simulink model "roachf_2048_bak"  (Simulink 7.5)
settings: Solver=ode45  StartTime=0.0  StopTime=10.0  RelTol=1e-3
undefined symbols (not defined in the file or in its folder): Down, quant, ROACH, Up
libraries: xbsIndex_r4 x1733, xps_library x50, casper_library_misc x29, casper_library_delays x4, casper_library_sources x4, xbsReplacements_r4 x4, simulink x1, xrbsControl_r4 x1
(part 1 of 18)
settings Model: Creator=zaki  LastModifiedBy=zaki
settings DebuggingCC: StrictBusMsg=Warning

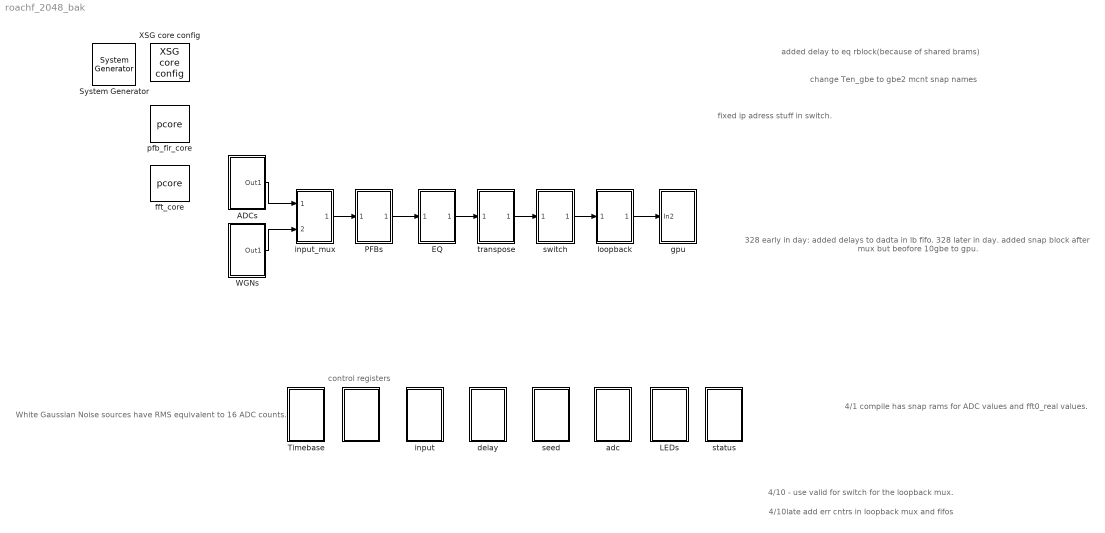
== system roachf_2048_bak ==
  note: White Gaussian Noise
      | sources have RMS
      | equivalent to 16 ADC
      | counts.
  note: fixed ip adress stuff in switch.
  note: 328 early in day: added delays to dadta in lb fifo.
      | 328 later in day. added snap block after mux but beofore
      | 10gbe to gpu.
  note: 4/1 compile has snap rams for ADC values
      | and fft0_real values.
  note: 4/10 - use valid for switch for the loopback mux.
  note: 4/10late add err cntrs in loopback mux and fifos
  note: control registers
  note: added delay to eq rblock(because of shared brams)
  note: change Ten_gbe to gbe2
      | mcnt snap names
  " " : Subsystem
  " System Generator" : xbsIndex_r4/ System Generator  Tag=genX  SystemSampleTime=-1  xilinxfamily=virtex5  part=xc5vsx95t  speed=-1  package=ff1136  synthesis_tool=XST  clock_wrapper=Clock Enables  directory=./roachf_2048_bak/sysgen  proj_type=Project Navigator  Synth_file=XST Defaults  Impl_file=ISE Defaults  testbench=off  simulink_period=1  sysclk_period=5  dcm_input_clock_period=100  incr_netlist=off  trim_vbits=Everywhere in SubSystem  dbl_ovrd=According to Block Masks  core_generation=According to Block Masks  run_coregen=off  deprecated_control=off  eval_field=0  block_type=sysgen
      settings: block_version=10.1.3
  ADCs : Subsystem
  EQ : Subsystem
  LEDs : Subsystem
  PFBs : Subsystem
  Timebase : Subsystem
  WGNs : Subsystem
  XSG core config : xps_library/XSG core config  Tag=xps:xsg  SystemSampleTime=-1  hw_sys=ROACH:sx95t  ibob_linux=off  clk_src=adc0_clk  iBOB_clk_src=adc0_clk  BEE2_ctrl_clk_src=sys_clk  BEE2_usr_clk_src=sys_clk  ROACH_clk_src=adc0_clk  ROACH2_clk_src=adc0_clk  CORR_clk_src=sys_clk  gpio_clk_io_group=iBOB:sma  gpio_clk_bit_index=0  clk_rate=200  sample_period=1  synthesis_tool=XST  mpc_type=powerpc440_ext
  adc : Subsystem
  delay : Subsystem
      settings: AttributesFormatString=|
      | Addr 8195
      | 0x2003
  fft_core : xps_library/pcore  Tag=xps:pcore  SystemSampleTime=-1  pcore_path=fft_core/fft_core.ngc
  gpu : Subsystem
  input : Subsystem
      settings: AttributesFormatString=|
      | Addr 8194
      | 0x2002
  input_mux : Subsystem
  loopback : Subsystem
  pfb_fir_core : xps_library/pcore  Tag=xps:pcore  SystemSampleTime=-1  pcore_path=pfb_fir_core/pfb_fir_core.ngc
  seed : Subsystem
      settings: AttributesFormatString=|
      | Addr 8196
      | 0x2004
  status : Subsystem
  switch : Subsystem
  transpose : Subsystem
  wires:
  ADCs/1(Out1) -> input_mux/1(In1)
  WGNs/1(Out1) -> input_mux/2(In2)
  input_mux/1(Out1) -> PFBs/1(In1)
  PFBs/1(Out1) -> EQ/1(In1)
  EQ/1(Out1) -> transpose/1(In1)
  transpose/1(Out1) -> switch/1(In2)
  switch/1(Out1) -> loopback/1(In1)
  loopback/1(Out1) -> gpu/1(In2)

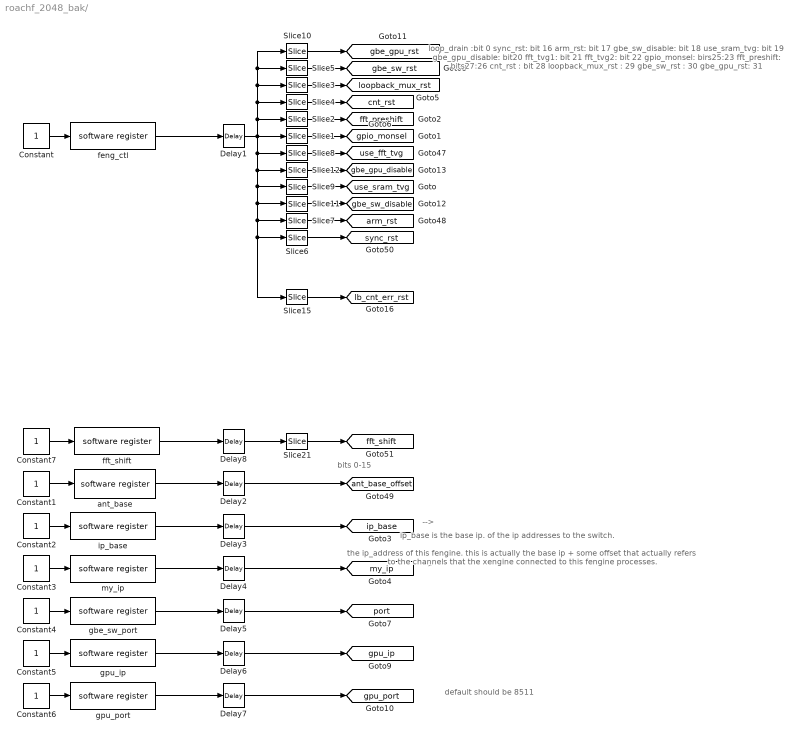
== system roachf_2048_bak/  ==
  note: bits 0-15
  note: default should be 8511
  note: -->
  note: -->
  note: the ip_address of this fengine.
      | this is actually the base ip + some offset
      | that actually refers to the channels that
      | the xengine connected to this fengine
      | processes.
  note: ip_base is the base ip. of
      | the ip addresses to the switch.
  note: loop_drain :bit 0
      |
      | sync_rst: bit 16
      | arm_rst: bit 17
      | gbe_sw_disable: bit 18
      | use_sram_tvg: bit 19
      | gbe_gpu_disable: bit20
      | fft_tvg1: bit 21
      | fft_tvg2: bit 22
      | gpio_monsel: birs25:23
      | fft_preshift: bits27:26
      | cnt_rst : bit 28
      | loopback_mux_rst : 29
      | gbe_sw_rst : 30
      | gbe_gpu_rst: 31
  Constant{,1..7} (8 alike) : Constant
  Delay{1..8} (8 alike) : xbsIndex_r4/Delay  rst=off  infoeditControl=Selection of Reset will increase slice count due to use of real FFs and instead of SRLs  en=off  latency=1  dbl_ovrd=off  reg_retiming=on  block_type=delay
      settings: xl_area=[0, 0, 0, 0, 0, 0, 0]
  Slice1 : xbsIndex_r4/Slice  nbits=3  boolean_output=off  mode=Lower Bit Location + Width  bit1=0  base1=MSB of Input  bit0=23  base0=LSB of Input  dbl_ovrd=off  block_type=slice
      settings: infoedit="Extracts a given range of bits from each input sample and presents it at the output.  The output type is ordinarily unsigned with binary point at zero, but can be Boolean when the slice is one bit wide.<P><P>Hardware notes: In hardware this block costs nothing."  block_version=10.1.3
  Slice10 : xbsIndex_r4/Slice  nbits=16  boolean_output=on  mode=Lower Bit Location + Width  bit1=0  base1=MSB of Input  bit0=31  base0=LSB of Input  dbl_ovrd=off  block_type=slice
      settings: block_version=10.1.3
  Slice11 : xbsIndex_r4/Slice  nbits=1  boolean_output=on  mode=Lower Bit Location + Width  bit1=0  base1=MSB of Input  bit0=18  base0=LSB of Input  dbl_ovrd=off  block_type=slice
      settings: block_version=10.1.3
  Slice12 : xbsIndex_r4/Slice  nbits=1  boolean_output=on  mode=Lower Bit Location + Width  bit1=0  base1=MSB of Input  bit0=20  base0=LSB of Input  dbl_ovrd=off  block_type=slice
      settings: block_version=10.1.3
  Slice15 : xbsIndex_r4/Slice  nbits=1  boolean_output=on  mode=Lower Bit Location + Width  bit1=0  base1=MSB of Input  bit0=2  base0=LSB of Input  dbl_ovrd=off  block_type=slice
      settings: block_version=10.1.3
  Slice2 : xbsIndex_r4/Slice  nbits=2  boolean_output=off  mode=Lower Bit Location + Width  bit1=0  base1=MSB of Input  bit0=26  base0=LSB of Input  dbl_ovrd=off  block_type=slice
      settings: infoedit="Extracts a given range of bits from each input sample and presents it at the output.  The output type is ordinarily unsigned with binary point at zero, but can be Boolean when the slice is one bit wide.<P><P>Hardware notes: In hardware this block costs nothing."  block_version=10.1.3
  Slice21 : xbsIndex_r4/Slice  nbits=16  boolean_output=off  mode=Lower Bit Location + Width  bit1=0  base1=MSB of Input  bit0=0  base0=LSB of Input  dbl_ovrd=off  block_type=slice
      settings: infoedit="Extracts a given range of bits from each input sample and presents it at the output.  The output type is ordinarily unsigned with binary point at zero, but can be Boolean when the slice is one bit wide.<P><P>Hardware notes: In hardware this block costs nothing."  block_version=10.1.3
  Slice3 : xbsIndex_r4/Slice  nbits=16  boolean_output=on  mode=Lower Bit Location + Width  bit1=0  base1=MSB of Input  bit0=29  base0=LSB of Input  dbl_ovrd=off  block_type=slice
      settings: block_version=10.1.3
  Slice4 : xbsIndex_r4/Slice  nbits=16  boolean_output=on  mode=Lower Bit Location + Width  bit1=0  base1=MSB of Input  bit0=28  base0=LSB of Input  dbl_ovrd=off  block_type=slice
      settings: block_version=10.1.3
  Slice5 : xbsIndex_r4/Slice  nbits=16  boolean_output=on  mode=Lower Bit Location + Width  bit1=0  base1=MSB of Input  bit0=30  base0=LSB of Input  dbl_ovrd=off  block_type=slice
      settings: block_version=10.1.3
  Slice6 : xbsIndex_r4/Slice  nbits=1  boolean_output=on  mode=Lower Bit Location + Width  bit1=0  base1=MSB of Input  bit0=16  base0=LSB of Input  dbl_ovrd=off  block_type=slice
      settings: infoedit="Extracts a given range of bits from each input sample and presents it at the output.  The output type is ordinarily unsigned with binary point at zero, but can be Boolean when the slice is one bit wide.<P><P>Hardware notes: In hardware this block costs nothing."  block_version=10.1.3
  Slice7 : xbsIndex_r4/Slice  nbits=1  boolean_output=on  mode=Lower Bit Location + Width  bit1=0  base1=MSB of Input  bit0=17  base0=LSB of Input  dbl_ovrd=off  block_type=slice
      settings: infoedit="Extracts a given range of bits from each input sample and presents it at the output.  The output type is ordinarily unsigned with binary point at zero, but can be Boolean when the slice is one bit wide.<P><P>Hardware notes: In hardware this block costs nothing."  block_version=10.1.3
  Slice8 : xbsIndex_r4/Slice  nbits=2  boolean_output=off  mode=Lower Bit Location + Width  bit1=0  base1=MSB of Input  bit0=21  base0=LSB of Input  dbl_ovrd=off  block_type=slice
      settings: infoedit="Extracts a given range of bits from each input sample and presents it at the output.  The output type is ordinarily unsigned with binary point at zero, but can be Boolean when the slice is one bit wide.<P><P>Hardware notes: In hardware this block costs nothing."  block_version=10.1.3
  Slice9 : xbsIndex_r4/Slice  nbits=1  boolean_output=off  mode=Lower Bit Location + Width  bit1=0  base1=MSB of Input  bit0=19  base0=LSB of Input  dbl_ovrd=off  block_type=slice
      settings: infoedit="Extracts a given range of bits from each input sample and presents it at the output.  The output type is ordinarily unsigned with binary point at zero, but can be Boolean when the slice is one bit wide.<P><P>Hardware notes: In hardware this block costs nothing."  block_version=10.1.3
  ant_base : xps_library/software register  Tag=xps:sw_reg  SystemSampleTime=-1  io_dir=From Processor  arith_type=Unsigned  bitwidth=32  bin_pt=0  sample_period=1  gw_name=roachf_2048_bak_ant_base_user_data_out
  feng_ctl : xps_library/software register  Tag=xps:sw_reg  SystemSampleTime=-1  io_dir=From Processor  arith_type=Unsigned  bitwidth=32  bin_pt=0  sample_period=1  gw_name=roachf_2048_bak_feng_ctl_user_data_out
  fft_shift : xps_library/software register  Tag=xps:sw_reg  SystemSampleTime=-1  io_dir=From Processor  arith_type=Unsigned  bitwidth=32  bin_pt=0  sample_period=1  gw_name=roachf_2048_bak_fft_shift_user_data_out
  gbe_sw_port : xps_library/software register  Tag=xps:sw_reg  SystemSampleTime=-1  io_dir=From Processor  arith_type=Unsigned  bitwidth=32  bin_pt=0  sample_period=1  gw_name=roachf_2048_bak_gbe_sw_port_user_data_out
  gpu_ip : xps_library/software register  Tag=xps:sw_reg  SystemSampleTime=-1  io_dir=From Processor  arith_type=Unsigned  bitwidth=32  bin_pt=0  sample_period=1  gw_name=roachf_2048_bak_gpu_ip_user_data_out
  gpu_port : xps_library/software register  Tag=xps:sw_reg  SystemSampleTime=-1  io_dir=From Processor  arith_type=Unsigned  bitwidth=32  bin_pt=0  sample_period=1  gw_name=roachf_2048_bak_gpu_port_user_data_out
  ip_base : xps_library/software register  Tag=xps:sw_reg  SystemSampleTime=-1  io_dir=From Processor  arith_type=Unsigned  bitwidth=32  bin_pt=0  sample_period=1  gw_name=roachf_2048_bak_ip_base_user_data_out
  my_ip : xps_library/software register  Tag=xps:sw_reg  SystemSampleTime=-1  io_dir=From Processor  arith_type=Unsigned  bitwidth=32  bin_pt=0  sample_period=1  gw_name=roachf_2048_bak_my_ip_user_data_out
  tag scope: ant_base_offset=global, arm_rst=global, cnt_rst=global, fft_preshift=global, fft_shift=global, gbe_gpu_disable=global, gbe_gpu_rst=global, gbe_sw_disable=global, gbe_sw_rst=global, gpio_monsel=global, gpu_ip=global, gpu_port=global, ip_base=global, lb_cnt_err_rst=global, loopback_mux_rst=global, my_ip=global, port=global, sync_rst=global, use_fft_tvg=global, use_sram_tvg=global
  wires:
  Slice15/1 -> [lb_cnt_err_rst]
  Delay8/1 -> Slice21/1
  Constant7/1 -> fft_shift/1
  fft_shift/1 -> Delay8/1
  Slice12/1 -> [gbe_gpu_disable]
  Slice11/1 -> [gbe_sw_disable]
  Slice10/1 -> [gbe_gpu_rst]
  gpu_port/1 -> Delay7/1
  Delay7/1 -> [gpu_port]
  Constant6/1 -> gpu_port/1
  gpu_ip/1 -> Delay6/1
  Delay6/1 -> [gpu_ip]
  Constant5/1 -> gpu_ip/1
  Slice5/1 -> [gbe_sw_rst]
  gbe_sw_port/1 -> Delay5/1
  Delay5/1 -> [port]
  Constant4/1 -> gbe_sw_port/1
  Slice3/1 -> [loopback_mux_rst]
  Slice4/1 -> [cnt_rst]
  my_ip/1 -> Delay4/1
  Delay4/1 -> [my_ip]
  Constant3/1 -> my_ip/1
  ip_base/1 -> Delay3/1
  Delay3/1 -> [ip_base]
  Constant2/1 -> ip_base/1
  Constant1/1 -> ant_base/1
  Constant/1 -> feng_ctl/1
  feng_ctl/1 -> Delay1/1
  Slice2/1 -> [fft_preshift]
  Slice1/1 -> [gpio_monsel]
  Slice9/1 -> [use_sram_tvg]
  Slice7/1 -> [arm_rst]
  Slice6/1 -> [sync_rst]
  Slice21/1 -> [fft_shift]
  Delay1/1 -> Slice10/1, Slice5/1, Slice3/1, Slice4/1, Slice2/1, Slice1/1, Slice12/1, Slice9/1, Slice11/1, Slice15/1, Slice6/1, Slice7/1, Slice8/1
  Slice8/1 -> [use_fft_tvg]
  ant_base/1 -> Delay2/1
  Delay2/1 -> [ant_base_offset]

== system roachf_2048_bak/ADCs ==
  Band-Limited White Noise : simulink/Sources/Band-Limited White Noise  SystemSampleTime=-1  Cov=[0.1]  Ts=0.1  seed=[23341]
  Constant : Constant  Value=0
  Constant1 : Constant
  Constant2 : Constant  Value=0
  Mux : Mux  Inputs=2
  Pulse Generator : DiscretePulseGenerator  Amplitude=1  Period=2^27  PulseWidth=1  PhaseDelay=0  SampleTime=1
  Terminator11 : Terminator
  Terminator12 : Terminator
  quadc0 : xps_library/quadc  Tag=xps:quadc  SystemSampleTime=-1  adc_brd=0  adc_clk_rate=200
  quadc1 : xps_library/quadc  Tag=xps:quadc  SystemSampleTime=-1  adc_brd=1  adc_clk_rate=200
  Out1 : Outport
  tag scope: adc0=global, adc1=global, adc2=global, adc3=global, adc4=global, adc5=global, adc6=global, adc7=global, sync_adc0=global, sync_adc1=global
  wires:
  quadc0/1 -> [adc0]
  quadc0/2 -> [adc1]
  quadc0/3 -> [adc2]
  quadc0/4 -> [adc3]
  quadc0/6 -> [sync_adc0]
  quadc1/1 -> [adc4]
  quadc1/2 -> [adc5]
  quadc1/3 -> [adc6]
  quadc1/4 -> [adc7]
  quadc1/6 -> [sync_adc1]
  Band-Limited White Noise/1 -> quadc1/4, quadc1/3, quadc1/2, quadc1/1, quadc0/4, quadc0/3, quadc0/2, quadc0/1
  Pulse Generator/1 -> quadc0/6, quadc1/6
  Constant1/1 -> quadc0/5, quadc1/5
  quadc0/5 -> Terminator11/1
  quadc1/5 -> Terminator12/1
  Mux/1 -> Out1/1
  Constant/1 -> Mux/1
  Constant2/1 -> Mux/2

== system roachf_2048_bak/EQ ==
  note: Next, we reduce the bitwidth out of the FFT to 4 bits to save resources.
      | This must be done very carefully to avoid losing too much information.
      | Firstly, this conversion is done using "Round to Even" and saturation
      | logic to avoid introducing DC offsets, wrapping, and other ugly quantizaton
      | effects.  Secondly, the "equalizer" allows each frequency channel of the
      | FFT to be scaled by a different number.  This allows passbands which are
      | not flat to be flattened digitally to allow for optimal quantization to 4 bits.
      | These coefficients can also be updated dynamically for gain control.
      | Coefficients can be dynamically written into the "equalizer" by
      | specifying the channel ("coeff_addr") and the value of the
      | coefficient ("coeff"), and then using "coeff_en" to write the
      | new coefficient into the equalization table.
  note: eq_mon will monitor eq0, which consists of
      | in0 spectrum followed by in1 spectrum
  In1 : Inport
  Concat3 : xbsIndex_r4/Concat  num_inputs=4  dbl_ovrd=off  block_type=concat
      settings: block_version=10.1.3
  Logical5 : xbsIndex_r4/Logical  logical_function=OR  inputs=4  en=off  latency=0  precision=Full  arith_type=Unsigned  n_bits=8  bin_pt=2  align_bp=on  dbl_ovrd=off  block_type=logical
      settings: xl_area=[0, 0, 0, 0, 0, 0, 0]
  Terminator13 : Terminator
  Terminator14 : Terminator
  Terminator15 : Terminator
  eq0 : Subsystem
  eq1 : Subsystem
  eq2 : Subsystem
  eq3 : Subsystem
  Out1 : Outport
  tag scope: eq0=global, eq1=global, eq2=global, eq3=global, eq_clip0=global, eq_clip1=global, eq_clip2=global, eq_clip3=global, eq_clip=global, eq_mon=global, eq_out=global, sync_rnd=global
  wires:
  eq0/1(dout) -> [eq0], [eq_mon]
  eq0/2(sync_out) -> [sync_rnd]
  [fft0] -> eq0/1(in)
  eq0/3(clip) -> [eq_clip0]
  Logical5/1 -> [eq_clip]
  Concat3/1 -> [eq_out]
  [eq_clip0] -> Logical5/1
  [eq_clip1] -> Logical5/2
  [fft1] -> eq1/1(in)
  [sync_fft0] -> eq1/2(sync)
  [fft2] -> eq2/1(in)
  [fft3] -> eq3/1(in)
  [sync_fft0] -> eq3/2(sync)
  eq1/1(dout) -> [eq1]
  eq1/2(sync_out) -> Terminator13/1
  eq1/3(clip) -> [eq_clip1]
  eq2/1(dout) -> [eq2]
  eq2/2(sync_out) -> Terminator14/1
  eq2/3(clip) -> [eq_clip2]
  eq3/1(dout) -> [eq3]
  eq3/2(sync_out) -> Terminator15/1
  eq3/3(clip) -> [eq_clip3]
  [eq_clip2] -> Logical5/3
  [eq_clip3] -> Logical5/4
  [eq0] -> Concat3/1
  [eq1] -> Concat3/2
  [eq2] -> Concat3/3
  [eq3] -> Concat3/4
  In1/1 -> Out1/1
  [sync_fft0] -> eq0/2(sync)
  [sync_fft0] -> eq2/2(sync)

== system roachf_2048_bak/LEDs ==
  Delay{,1..7} (8 alike) : xbsIndex_r4/Delay  rst=off  infoeditControl=Selection of Reset will increase slice count due to use of real FFs and instead of SRLs  en=off  latency=1  dbl_ovrd=off  reg_retiming=off  block_type=delay
  Terminator{1..3,27} (4 alike) : Terminator
  led1_arm : xps_library/gpio  Tag=xps:gpio  SystemSampleTime=-1  io_group=ROACH:led  io_dir=out  arith_type=Boolean  bitwidth=1  bin_pt=0  bit_index=0  sample_period=1  use_single_ended=off  use_ddr=off  reg_iob=on  reg_clk_phase=0  termination=None
      link override convert: arith_type=Boolean  n_bits=1  bin_pt=0
  led2_sync : xps_library/gpio  Tag=xps:gpio  SystemSampleTime=-1  io_group=ROACH:led  io_dir=out  arith_type=Boolean  bitwidth=1  bin_pt=0  bit_index=1  sample_period=1  use_single_ended=off  use_ddr=off  reg_iob=on  reg_clk_phase=0  termination=None
      link override convert: arith_type=Boolean  n_bits=1  bin_pt=0
  led4_eq_clip : xps_library/gpio  Tag=xps:gpio  SystemSampleTime=-1  io_group=ROACH:led  io_dir=out  arith_type=Boolean  bitwidth=1  bin_pt=0  bit_index=2  sample_period=1  use_single_ended=off  use_ddr=off  reg_iob=on  reg_clk_phase=0  termination=None
      link override convert: arith_type=Boolean  n_bits=1  bin_pt=0
  led5_adc_clip : xps_library/gpio  Tag=xps:gpio  SystemSampleTime=-1  io_group=ROACH:led  io_dir=out  arith_type=Boolean  bitwidth=1  bin_pt=0  bit_index=3  sample_period=1  use_single_ended=off  use_ddr=off  reg_iob=on  reg_clk_phase=0  termination=None
      link override convert: arith_type=Boolean  n_bits=1  bin_pt=0
  pulse_ext2 : Subsystem  mask{pulse_len=2^23}
      mask prompts: pulse_len "Length of Pulse"
      mask init:
      | bits = ceil(log2(pulse_len+1));
  pulse_ext3 : Subsystem  mask{pulse_len=2^18}
      mask prompts: pulse_len "Length of Pulse"
      mask init:
      | bits = ceil(log2(pulse_len+1));
  pulse_ext4 : Subsystem  mask{pulse_len=2^18}
      mask prompts: pulse_len "Length of Pulse"
      mask init:
      | bits = ceil(log2(pulse_len+1));
  wires:
  pulse_ext2/1(out) -> Delay5/1
  [sync_gen] -> Delay1/1
  [armed] -> Delay/1
  pulse_ext4/1(out) -> Delay6/1
  [eq_clip] -> Delay2/1
  led1_arm/1 -> Terminator27/1
  led4_eq_clip/1 -> Terminator2/1
  led2_sync/1 -> Terminator3/1
  [adc_clip] -> Delay3/1
  pulse_ext3/1(out) -> Delay7/1
  led5_adc_clip/1 -> Terminator1/1
  Delay/1 -> Delay4/1
  Delay3/1 -> pulse_ext3/1(in)
  Delay2/1 -> pulse_ext4/1(in)
  Delay1/1 -> pulse_ext2/1(in)
  Delay7/1 -> led5_adc_clip/1
  Delay6/1 -> led4_eq_clip/1
  Delay5/1 -> led2_sync/1
  Delay4/1 -> led1_arm/1

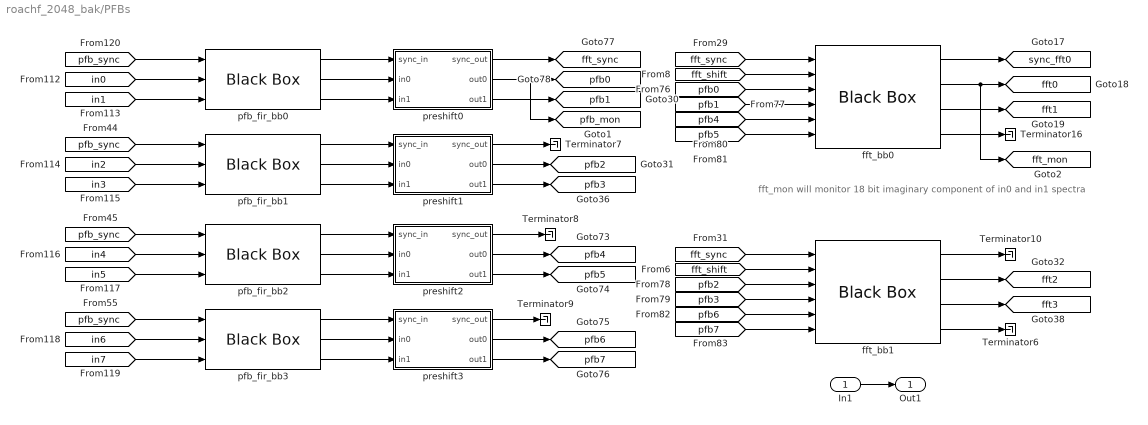
== system roachf_2048_bak/PFBs ==
  note: fft_mon will monitor 18 bit imaginary component of in0 and in1 spectra
  In1 : Inport
  Terminator{6..10,16} (6 alike) : Terminator
  fft_bb0 : xbsIndex_r4/Black Box  init_code=fft_core_config  sim_method=Inactive  verbose=off  block_type=blackbox2
      settings: infoedit="Incorporates black box HDL and simulation model into a System Generator design.<P><P>You must supply a Black Box with certain information about the HDL component you would like to bring into System Generator. This information is provided through a Matlab function.<P><P>When \"Simulation mode\" is set to \"Inactive\", you will typically want to provide a separate simulation model by using a Simulation Multiplexer.<P>When \"Simulation mode\" is set to \"External co-simulator\", you must include a ModelSim block in the design."  block_version=10.1.3
  fft_bb1 : xbsIndex_r4/Black Box  init_code=fft_core_config  sim_method=Inactive  verbose=off  block_type=blackbox2
      settings: infoedit="Incorporates black box HDL and simulation model into a System Generator design.<P><P>You must supply a Black Box with certain information about the HDL component you would like to bring into System Generator. This information is provided through a Matlab function.<P><P>When \"Simulation mode\" is set to \"Inactive\", you will typically want to provide a separate simulation model by using a Simulation Multiplexer.<P>When \"Simulation mode\" is set to \"External co-simulator\", you must include a ModelSim block in the design."  block_version=10.1.3
  pfb_fir_bb{0..3} (4 alike) : xbsIndex_r4/Black Box  init_code=pfb_fir_core_config  sim_method=Inactive  verbose=off  block_type=blackbox2
      settings: infoedit="Incorporates black box HDL and simulation model into a System Generator design.<P><P>You must supply a Black Box with certain information about the HDL component you would like to bring into System Generator. This information is provided through a Matlab function.<P><P>When \"Simulation mode\" is set to \"Inactive\", you will typically want to provide a separate simulation model by using a Simulation Multiplexer.<P>When \"Simulation mode\" is set to \"External co-simulator\", you must include a ModelSim block in the design."  block_version=10.1.3
  preshift0 : Subsystem
  preshift1 : Subsystem
  preshift2 : Subsystem
  preshift3 : Subsystem
  Out1 : Outport
  tag scope: fft0=global, fft1=global, fft2=global, fft3=global, fft_mon=global, fft_sync=global, pfb0=global, pfb1=global, pfb2=global, pfb3=global, pfb4=global, pfb5=global, pfb6=global, pfb7=global, pfb_mon=global, sync_fft0=global
  wires:
  [in0] -> pfb_fir_bb0/2
  [in1] -> pfb_fir_bb0/3
  [in2] -> pfb_fir_bb1/2
  [in3] -> pfb_fir_bb1/3
  [in4] -> pfb_fir_bb2/2
  [in5] -> pfb_fir_bb2/3
  [in6] -> pfb_fir_bb3/2
  [in7] -> pfb_fir_bb3/3
  pfb_fir_bb1/2 -> preshift1/2(in0)
  pfb_fir_bb1/3 -> preshift1/3(in1)
  pfb_fir_bb2/2 -> preshift2/2(in0)
  pfb_fir_bb2/3 -> preshift2/3(in1)
  pfb_fir_bb3/2 -> preshift3/2(in0)
  pfb_fir_bb3/3 -> preshift3/3(in1)
  [fft_sync] -> fft_bb1/1
  [fft_shift] -> fft_bb1/2
  fft_bb1/3 -> [fft3]
  fft_bb1/4 -> Terminator6/1
  fft_bb1/2 -> [fft2]
  fft_bb0/2 -> [fft0], [fft_mon]
  fft_bb0/3 -> [fft1]
  fft_bb0/4 -> Terminator16/1
  fft_bb0/1 -> [sync_fft0]
  fft_bb1/1 -> Terminator10/1
  [fft_sync] -> fft_bb0/1
  [fft_shift] -> fft_bb0/2
  [pfb_sync] -> pfb_fir_bb0/1
  [pfb_sync] -> pfb_fir_bb1/1
  [pfb_sync] -> pfb_fir_bb2/1
  [pfb_sync] -> pfb_fir_bb3/1
  pfb_fir_bb1/1 -> preshift1/1(sync_in)
  pfb_fir_bb2/1 -> preshift2/1(sync_in)
  pfb_fir_bb3/1 -> preshift3/1(sync_in)
  In1/1 -> Out1/1
  pfb_fir_bb0/1 -> preshift0/1(sync_in)
  pfb_fir_bb0/2 -> preshift0/2(in0)
  pfb_fir_bb0/3 -> preshift0/3(in1)
  preshift0/1(sync_out) -> [fft_sync]
  preshift0/2(out0) -> [pfb0], [pfb_mon]
  preshift0/3(out1) -> [pfb1]
  preshift1/1(sync_out) -> Terminator7/1
  preshift2/1(sync_out) -> Terminator8/1
  preshift3/1(sync_out) -> Terminator9/1
  preshift1/2(out0) -> [pfb2]
  preshift1/3(out1) -> [pfb3]
  preshift2/2(out0) -> [pfb4]
  preshift2/3(out1) -> [pfb5]
  preshift3/2(out0) -> [pfb6]
  preshift3/3(out1) -> [pfb7]
  [pfb0] -> fft_bb0/3
  [pfb1] -> fft_bb0/4
  [pfb4] -> fft_bb0/5
  [pfb5] -> fft_bb0/6
  [pfb2] -> fft_bb1/3
  [pfb3] -> fft_bb1/4
  [pfb6] -> fft_bb1/5
  [pfb7] -> fft_bb1/6

== system roachf_2048_bak/Timebase ==
  note: This circuit implements a scheme for synchronizing
      | multiple IBOBs to within a 200 MHz clock.  "arm_rst"
      | comes from the "ctrl" register, and indicates that the
      | next "sync_rst" should reset the sync pulse generated
      | in "sync_gen".  "sync_rst" may be generated from
      | the "ctrl" register, but more often, a 1PPS signal is
      | supplied to the "sync" input of an ADC for this
      | purpose.  Once a reset occurs, "sync_gen" generates
      | a single pulse every 2^27 clocks.  LEDs provide visual
      | confirmation that an arm and reset occur, and "mrst"
      | is passed over XAUI for the purpose of informing
      | downstream logic in a packetized correlator that
      | a "master reset" has occurred.
  Logical7 : xbsIndex_r4/Logical  logical_function=OR  inputs=3  en=off  latency=1  precision=Full  arith_type=Unsigned  n_bits=8  bin_pt=2  align_bp=on  dbl_ovrd=off  block_type=logical
      settings: xl_area=[0, 0, 0, 0, 0, 0, 0]
  sync_gen : Subsystem
  tag scope: armed=global, mrst=global, sync_gen=global
  wires:
  sync_gen/1(sync) -> [sync_gen]
  sync_gen/2(mrst) -> [mrst]
  [arm_rst] -> sync_gen/2(arm)
  [sync_rst] -> Logical7/2
  sync_gen/3(armed) -> [armed]
  Logical7/1 -> sync_gen/1(rst)
  [sync_adc0] -> Logical7/1
  [sync_adc1] -> Logical7/3

== system roachf_2048_bak/WGNs ==
  Constant : Constant  Value=0
  Constant2 : Constant  Value=0
  Mux : Mux  Inputs=2
  noise0 : Subsystem
  noise1 : Subsystem
  noise2 : Subsystem
  noise3 : Subsystem
  Out1 : Outport
  tag scope: n00=global, n01=global, n10=global, n11=global, n20=global, n21=global, n30=global, n31=global
  wires:
  noise0/1(n1) -> [n00]
  noise0/2(n2) -> [n01]
  noise1/1(n1) -> [n10]
  noise1/2(n2) -> [n11]
  noise2/1(n1) -> [n20]
  noise2/2(n2) -> [n21]
  noise3/1(n1) -> [n30]
  noise3/2(n2) -> [n31]
  [seed0] -> noise0/1(seed)
  [seed3] -> noise3/1(seed)
  [seed2] -> noise2/1(seed)
  [seed1] -> noise1/1(seed)
  [seed_ld] -> noise0/2(ld)
  [seed_ld] -> noise3/2(ld)
  [seed_ld] -> noise2/2(ld)
  [seed_ld] -> noise1/2(ld)
  Mux/1 -> Out1/1
  Constant/1 -> Mux/1
  Constant2/1 -> Mux/2

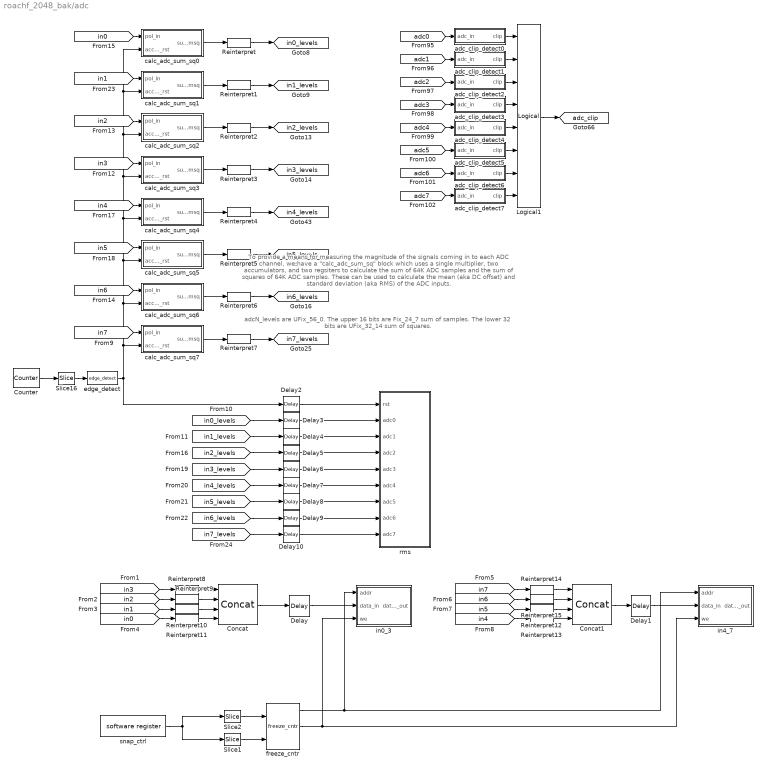
== system roachf_2048_bak/adc ==
  note: To provide a means for measuring the magnitude of the signals
      | coming in to each ADC channel, we have a "calc_adc_sum_sq"
      | block which uses a single multiplier, two accumulators, and two
      | regsiters to calculate the sum of 64K ADC samples and the sum
      | of squares of 64K ADC samples.  These can be used to calculate
      | the mean (aka DC offset) and standard deviation (aka RMS) of
      | the ADC inputs.
  note: adcN_levels are UFix_56_0.
      | The upper 16 bits are Fix_24_7 sum of samples.
      | The lower 32 bits are UFix_32_14 sum of squares.
  Concat : xbsIndex_r4/Concat  num_inputs=4  dbl_ovrd=off  block_type=concat
  Concat1 : xbsIndex_r4/Concat  num_inputs=4  dbl_ovrd=off  block_type=concat
  Counter : xbsIndex_r4/Counter  cnt_type=Free Running  cnt_to=Inf  operation=Up  start_count=0  cnt_by_val=1  arith_type=Unsigned  n_bits=16  bin_pt=0  load_pin=off  rst=off  en=off  explicit_period=off  period=1  dbl_ovrd=off  use_behavioral_HDL=off  implementation=Fabric  use_rpm=on  block_type=counter
      settings: xl_area=[0, 0, 0, 0, 0, 0, 0]
  Delay : xbsIndex_r4/Delay  rst=off  infoeditControl=Selection of Reset will increase slice count due to use of real FFs and instead of SRLs  en=off  latency=3  dbl_ovrd=off  reg_retiming=off  block_type=delay
  Delay1 : xbsIndex_r4/Delay  rst=off  infoeditControl=Selection of Reset will increase slice count due to use of real FFs and instead of SRLs  en=off  latency=3  dbl_ovrd=off  reg_retiming=off  block_type=delay
  Delay{2..10} (9 alike) : xbsIndex_r4/Delay  rst=off  infoeditControl=Selection of Reset will increase slice count due to use of real FFs and instead of SRLs  en=off  latency=2  dbl_ovrd=off  reg_retiming=off  block_type=delay
  Logical1 : xbsIndex_r4/Logical  logical_function=OR  inputs=8  en=off  latency=1  precision=Full  arith_type=Unsigned  n_bits=8  bin_pt=2  align_bp=on  dbl_ovrd=off  block_type=logical
      settings: xl_area=[0, 0, 0, 0, 0, 0, 0]
  Reinterpret{,1..15} (16 alike) : xbsIndex_r4/Reinterpret  force_arith_type=on  arith_type=Unsigned  force_bin_pt=on  bin_pt=0  block_type=reinterpret
      Reinterpret settings: infoedit="Changes signal type without altering the binary representation.   You can changed the signal between signed and unsigned, and relocate the binary point.<P><P>Hardware notes: In hardware this block costs nothing.<P><P>Example:  Suppose the input is 6 bits wide, signed, with 2 fractional bits, and the output is forced to unsigned with 0 fractional bits.  Then an input of -2.0 (1110.00 in binary 2's complement) becomes an output of 56 (111000 in binary)."  block_version=10.1.3
      Reinterpret1 settings: infoedit="Changes signal type without altering the binary representation.   You can changed the signal between signed and unsigned, and relocate the binary point.<P><P>Hardware notes: In hardware this block costs nothing.<P><P>Example:  Suppose the input is 6 bits wide, signed, with 2 fractional bits, and the output is forced to unsigned with 0 fractional bits.  Then an input of -2.0 (1110.00 in binary 2's complement) becomes an output of 56 (111000 in binary)."  block_version=10.1.3
      Reinterpret10 settings: block_version=10.1.3
      Reinterpret11 settings: block_version=10.1.3
      Reinterpret12 settings: block_version=10.1.3
      Reinterpret13 settings: block_version=10.1.3
      Reinterpret14 settings: block_version=10.1.3
      Reinterpret15 settings: block_version=10.1.3
      Reinterpret2 settings: infoedit="Changes signal type without altering the binary representation.   You can changed the signal between signed and unsigned, and relocate the binary point.<P><P>Hardware notes: In hardware this block costs nothing.<P><P>Example:  Suppose the input is 6 bits wide, signed, with 2 fractional bits, and the output is forced to unsigned with 0 fractional bits.  Then an input of -2.0 (1110.00 in binary 2's complement) becomes an output of 56 (111000 in binary)."  block_version=10.1.3
      Reinterpret3 settings: infoedit="Changes signal type without altering the binary representation.   You can changed the signal between signed and unsigned, and relocate the binary point.<P><P>Hardware notes: In hardware this block costs nothing.<P><P>Example:  Suppose the input is 6 bits wide, signed, with 2 fractional bits, and the output is forced to unsigned with 0 fractional bits.  Then an input of -2.0 (1110.00 in binary 2's complement) becomes an output of 56 (111000 in binary)."  block_version=10.1.3
      Reinterpret4 settings: infoedit="Changes signal type without altering the binary representation.   You can changed the signal between signed and unsigned, and relocate the binary point.<P><P>Hardware notes: In hardware this block costs nothing.<P><P>Example:  Suppose the input is 6 bits wide, signed, with 2 fractional bits, and the output is forced to unsigned with 0 fractional bits.  Then an input of -2.0 (1110.00 in binary 2's complement) becomes an output of 56 (111000 in binary)."  block_version=10.1.3
      Reinterpret5 settings: infoedit="Changes signal type without altering the binary representation.   You can changed the signal between signed and unsigned, and relocate the binary point.<P><P>Hardware notes: In hardware this block costs nothing.<P><P>Example:  Suppose the input is 6 bits wide, signed, with 2 fractional bits, and the output is forced to unsigned with 0 fractional bits.  Then an input of -2.0 (1110.00 in binary 2's complement) becomes an output of 56 (111000 in binary)."  block_version=10.1.3
      Reinterpret6 settings: infoedit="Changes signal type without altering the binary representation.   You can changed the signal between signed and unsigned, and relocate the binary point.<P><P>Hardware notes: In hardware this block costs nothing.<P><P>Example:  Suppose the input is 6 bits wide, signed, with 2 fractional bits, and the output is forced to unsigned with 0 fractional bits.  Then an input of -2.0 (1110.00 in binary 2's complement) becomes an output of 56 (111000 in binary)."  block_version=10.1.3
      Reinterpret7 settings: infoedit="Changes signal type without altering the binary representation.   You can changed the signal between signed and unsigned, and relocate the binary point.<P><P>Hardware notes: In hardware this block costs nothing.<P><P>Example:  Suppose the input is 6 bits wide, signed, with 2 fractional bits, and the output is forced to unsigned with 0 fractional bits.  Then an input of -2.0 (1110.00 in binary 2's complement) becomes an output of 56 (111000 in binary)."  block_version=10.1.3
      Reinterpret8 settings: block_version=10.1.3
      Reinterpret9 settings: block_version=10.1.3
  Slice1 : xbsIndex_r4/Slice  nbits=1  boolean_output=on  mode=Lower Bit Location + Width  bit1=0  base1=MSB of Input  bit0=0  base0=LSB of Input  dbl_ovrd=off  block_type=slice
      settings: block_version=10.1.3
  Slice16 : xbsIndex_r4/Slice  nbits=1  boolean_output=on  mode=Upper Bit Location + Width  bit1=0  base1=MSB of Input  bit0=19  base0=LSB of Input  dbl_ovrd=off  block_type=slice
      settings: infoedit="Extracts a given range of bits from each input sample and presents it at the output.  The output type is ordinarily unsigned with binary point at zero, but can be Boolean when the slice is one bit wide.<P><P>Hardware notes: In hardware this block costs nothing."  block_version=10.1.3
  Slice2 : xbsIndex_r4/Slice  nbits=1  boolean_output=on  mode=Lower Bit Location + Width  bit1=0  base1=MSB of Input  bit0=1  base0=LSB of Input  dbl_ovrd=off  block_type=slice
      settings: block_version=10.1.3
  adc_clip_detect0 : Subsystem  mask{n_bits=8}
      mask prompts: n_bits "Number of ADC bits"
  adc_clip_detect1 : Subsystem  mask{n_bits=8}
      mask prompts: n_bits "Number of ADC bits"
  adc_clip_detect2 : Subsystem  mask{n_bits=8}
      mask prompts: n_bits "Number of ADC bits"
  adc_clip_detect3 : Subsystem  mask{n_bits=8}
      mask prompts: n_bits "Number of ADC bits"
  adc_clip_detect4 : Subsystem  mask{n_bits=8}
      mask prompts: n_bits "Number of ADC bits"
  adc_clip_detect5 : Subsystem  mask{n_bits=8}
      mask prompts: n_bits "Number of ADC bits"
  adc_clip_detect6 : Subsystem  mask{n_bits=8}
      mask prompts: n_bits "Number of ADC bits"
  adc_clip_detect7 : Subsystem  mask{n_bits=8}
      mask prompts: n_bits "Number of ADC bits"
  calc_adc_sum_sq0 : Subsystem
  calc_adc_sum_sq1 : Subsystem
  calc_adc_sum_sq2 : Subsystem
  calc_adc_sum_sq3 : Subsystem
  calc_adc_sum_sq4 : Subsystem
  calc_adc_sum_sq5 : Subsystem
  calc_adc_sum_sq6 : Subsystem
  calc_adc_sum_sq7 : Subsystem
  edge_detect : casper_library_misc/edge_detect  SystemSampleTime=-1  edge=Rising  polarity=Active High  x_in=[0 0.08 0.08 0.16 0.16 0.24 0.24 0.32 0.32 0.4]  y_in="[-1 -1  1  1  1  1  1  1  1  1]"  x_out=[0.6 0.68 0.68 0.76 0.76 0.84 0.84 0.92 0.92 1]  y_out="[-1 -1  1  1 -1 -1 -1 -1 -1 -1]"
  freeze_cntr : casper_library_misc/freeze_cntr  SystemSampleTime=-1  CounterBits=10
  in0_3 : Subsystem  Tag=xps:bram  AncestorBlock=xps_library/Shared BRAM  mask{arith_type=Unsigned, addr_width=10, data_width=32, data_bin_pt=0, init_vals=[0:2^10-1], sample_rate=1}
      mask prompts: arith_type "Output Data Type"; addr_width "Address width"; data_width "Data Width"; data_bin_pt "Data Binary Point"; init_vals "Initial 32-bit values (simulation only)"; sample_rate "Sample rate"
      mask init:
      | shared_bram_mask;
      mask StyleString: popup(Unsigned|Signed  (2's comp)),edit,popup(8|16|32|64|128),edit,edit,edit
  in4_7 : Subsystem  Tag=xps:bram  AncestorBlock=xps_library/Shared BRAM  mask{arith_type=Unsigned, addr_width=10, data_width=32, data_bin_pt=0, init_vals=[0:2^10-1], sample_rate=1}
      mask prompts: arith_type "Output Data Type"; addr_width "Address width"; data_width "Data Width"; data_bin_pt "Data Binary Point"; init_vals "Initial 32-bit values (simulation only)"; sample_rate "Sample rate"
      mask init:
      | shared_bram_mask;
      mask StyleString: popup(Unsigned|Signed  (2's comp)),edit,popup(8|16|32|64|128),edit,edit,edit
  rms : Subsystem
  snap_ctrl : xps_library/software register  Tag=xps:sw_reg  SystemSampleTime=-1  io_dir=From Processor  arith_type=Unsigned  bitwidth=32  bin_pt=0  sample_period=1  gw_name=roachf_2048_bak_adc_snap_ctrl_user_data_out
  tag scope: adc_clip=global, in0_levels=global, in1_levels=global, in2_levels=global, in3_levels=global, in4_levels=global, in5_levels=global, in6_levels=global, in7_levels=global
  wires:
  [in0] -> calc_adc_sum_sq0/1(pol_in)
  calc_adc_sum_sq1/1(sum_sumsq) -> Reinterpret1/1
  calc_adc_sum_sq2/1(sum_sumsq) -> Reinterpret2/1
  calc_adc_sum_sq3/1(sum_sumsq) -> Reinterpret3/1
  edge_detect/1 -> calc_adc_sum_sq0/2(accum_rst), calc_adc_sum_sq1/2(accum_rst), calc_adc_sum_sq2/2(accum_rst), calc_adc_sum_sq3/2(accum_rst), calc_adc_sum_sq4/2(accum_rst), calc_adc_sum_sq5/2(accum_rst), calc_adc_sum_sq6/2(accum_rst), calc_adc_sum_sq7/2(accum_rst), Delay2/1
  [in1] -> calc_adc_sum_sq1/1(pol_in)
  [in2] -> calc_adc_sum_sq2/1(pol_in)
  [in3] -> calc_adc_sum_sq3/1(pol_in)
  Counter/1 -> Slice16/1
  Slice16/1 -> edge_detect/1
  calc_adc_sum_sq4/1(sum_sumsq) -> Reinterpret4/1
  [in4] -> calc_adc_sum_sq4/1(pol_in)
  calc_adc_sum_sq5/1(sum_sumsq) -> Reinterpret5/1
  calc_adc_sum_sq6/1(sum_sumsq) -> Reinterpret6/1
  calc_adc_sum_sq7/1(sum_sumsq) -> Reinterpret7/1
  [in5] -> calc_adc_sum_sq5/1(pol_in)
  [in6] -> calc_adc_sum_sq6/1(pol_in)
  [in7] -> calc_adc_sum_sq7/1(pol_in)
  Reinterpret/1 -> [in0_levels]
  Reinterpret1/1 -> [in1_levels]
  Reinterpret2/1 -> [in2_levels]
  Reinterpret3/1 -> [in3_levels]
  Reinterpret4/1 -> [in4_levels]
  Reinterpret5/1 -> [in5_levels]
  Reinterpret6/1 -> [in6_levels]
  Reinterpret7/1 -> [in7_levels]
  calc_adc_sum_sq0/1(sum_sumsq) -> Reinterpret/1
  [adc0] -> adc_clip_detect0/1(adc_in)
  [adc1] -> adc_clip_detect1/1(adc_in)
  [adc2] -> adc_clip_detect2/1(adc_in)
  [adc3] -> adc_clip_detect3/1(adc_in)
  [adc4] -> adc_clip_detect4/1(adc_in)
  [adc5] -> adc_clip_detect5/1(adc_in)
  [adc6] -> adc_clip_detect6/1(adc_in)
  [adc7] -> adc_clip_detect7/1(adc_in)
  adc_clip_detect0/1(clip) -> Logical1/1
  adc_clip_detect1/1(clip) -> Logical1/2
  adc_clip_detect2/1(clip) -> Logical1/3
  adc_clip_detect3/1(clip) -> Logical1/4
  adc_clip_detect4/1(clip) -> Logical1/5
  adc_clip_detect5/1(clip) -> Logical1/6
  adc_clip_detect6/1(clip) -> Logical1/7
  adc_clip_detect7/1(clip) -> Logical1/8
  Logical1/1 -> [adc_clip]
  [in3] -> Reinterpret8/1
  [in2] -> Reinterpret9/1
  [in1] -> Reinterpret10/1
  [in0] -> Reinterpret11/1
  Reinterpret8/1 -> Concat/1
  Reinterpret9/1 -> Concat/2
  Reinterpret10/1 -> Concat/3
  Reinterpret11/1 -> Concat/4
  Concat/1 -> Delay/1
  [in7] -> Reinterpret14/1
  [in6] -> Reinterpret15/1
  [in5] -> Reinterpret12/1
  [in4] -> Reinterpret13/1
  Reinterpret14/1 -> Concat1/1
  Reinterpret15/1 -> Concat1/2
  Reinterpret12/1 -> Concat1/3
  Reinterpret13/1 -> Concat1/4
  Concat1/1 -> Delay1/1
  freeze_cntr/1 -> in0_3/1(addr), in4_7/1(addr)
  freeze_cntr/2 -> in4_7/3(we), in0_3/3(we)
  Slice1/1 -> freeze_cntr/2
  Slice2/1 -> freeze_cntr/1
  snap_ctrl/1 -> Slice2/1, Slice1/1
  Delay/1 -> in0_3/2(data_in)
  Delay1/1 -> in4_7/2(data_in)
  [in0_levels] -> Delay3/1
  [in1_levels] -> Delay4/1
  [in2_levels] -> Delay5/1
  [in3_levels] -> Delay6/1
  [in4_levels] -> Delay7/1
  [in5_levels] -> Delay8/1
  [in6_levels] -> Delay9/1
  [in7_levels] -> Delay10/1
  Delay2/1 -> rms/1(rst)
  Delay3/1 -> rms/2(adc0)
  Delay4/1 -> rms/3(adc1)
  Delay5/1 -> rms/4(adc2)
  Delay6/1 -> rms/5(adc3)
  Delay7/1 -> rms/6(adc4)
  Delay8/1 -> rms/7(adc5)
  Delay9/1 -> rms/8(adc6)
  Delay10/1 -> rms/9(adc7)

== system roachf_2048_bak/delay ==
  BitBasher : xbsIndex_r4/BitBasher  bitexpr=|  display_expr=off  sr_1=1  arith_type1=Unsigned  bin_pt1=0  sr_2=2  arith_type2=Unsigned  bin_pt2=0  sr_3=3  arith_type3=Unsigned  bin_pt3=0  sr_4=4  arith_type4=Unsigned  bin_pt4=0  dbl_ovrd=off  block_type=bitbasher
      | <html><head><meta name="qrichtext" content="1" /><style type="text/css">
      | p, li { white-space: pre-wrap; }
      | </style></head><body style=" font-family:'MS Shell Dlg 2'; font-size:8.25pt; font-weight:400; font-style:normal;">
      | <p style=" margin-top:12px; margin-bottom:12px; margin-left:0px; margin-right:0px; -qt-block-indent:0; text-indent:0px; font-family:'MS Shell Dlg'; font-size:8pt;">delay0={delay[3:0]}<br />delay1={delay[7:4]}<br />delay2={delay[11:8]}<br />delay3={delay[15:12]}</p></body></html>
      settings: block_version=10.1.3
  BitBasher1 : xbsIndex_r4/BitBasher  bitexpr=|  display_expr=off  sr_1=1  arith_type1=Unsigned  bin_pt1=0  sr_2=2  arith_type2=Unsigned  bin_pt2=0  sr_3=3  arith_type3=Unsigned  bin_pt3=0  sr_4=4  arith_type4=Unsigned  bin_pt4=0  dbl_ovrd=off  block_type=bitbasher
      | <html><head><meta name="qrichtext" content="1" /><style type="text/css">
      | p, li { white-space: pre-wrap; }
      | </style></head><body style=" font-family:'MS Shell Dlg 2'; font-size:8.25pt; font-weight:400; font-style:normal;">
      | <p style=" margin-top:12px; margin-bottom:12px; margin-left:0px; margin-right:0px; -qt-block-indent:0; text-indent:0px; font-family:'MS Shell Dlg'; font-size:8pt;">delay4={delay[19:16]}<br />delay5={delay[23:20]}<br />delay6={delay[27:24]}<br />delay7={delay[31:28]}</p></body></html>
      settings: block_version=10.1.3
  values : xps_library/software register  Tag=xps:sw_reg  SystemSampleTime=-1  io_dir=From Processor  arith_type=Unsigned  bitwidth=32  bin_pt=0  sample_period=1  gw_name=roachf_2048_bak_delay_values_user_data_out
  tag scope: delay0=global, delay1=global, delay2=global, delay3=global, delay4=global, delay5=global, delay6=global, delay7=global
  wires:
  values/1 -> BitBasher1/1, BitBasher/1
  BitBasher1/4 -> [delay7]
  BitBasher1/3 -> [delay6]
  BitBasher1/2 -> [delay5]
  BitBasher1/1 -> [delay4]
  BitBasher/4 -> [delay3]
  BitBasher/3 -> [delay2]
  BitBasher/2 -> [delay1]
  BitBasher/1 -> [delay0]

== system roachf_2048_bak/gpu ==
  note: data comes in directly from the loopback musx into the ten gbe core
  In2 : Inport
  10gbetxsnap : Subsystem  mask{nsamples=11}
      mask prompts: nsamples "Number of samples (2^?)"
  Constant1 : xbsIndex_r4/Constant  const=0  gui_display_data_type=Fixed-point  arith_type=Boolean  n_bits=16  bin_pt=14  preci_type=Single  exp_width=8  frac_width=24  explicit_period=on  period=1  equ=P=C  opselect=C  inp2=PCIN>>17  opr=+  inp1=P  carry=CIN  dbl_ovrd=off  block_type=constant
      settings: block_version=11.4
  Constant2 : xbsIndex_r4/Constant  const=0  gui_display_data_type=Fixed-point  arith_type=Unsigned  n_bits=27  bin_pt=0  preci_type=Single  exp_width=8  frac_width=24  explicit_period=on  period=1  equ=P=C  opselect=C  inp2=PCIN>>17  opr=+  inp1=P  carry=CIN  dbl_ovrd=off  block_type=constant
      settings: block_version=11.4
  Delay{,1,3..5,10} (6 alike) : xbsIndex_r4/Delay  rst=off  infoeditControl=Selection of Reset will increase slice count due to use of real FFs and instead of SRLs  en=off  latency=2  dbl_ovrd=off  reg_retiming=off  block_type=delay
  Inverter : xbsIndex_r4/Inverter  en=off  latency=0  dbl_ovrd=off  block_type=inv
      settings: block_version=11.4
  Inverter1 : xbsIndex_r4/Inverter  en=off  latency=0  dbl_ovrd=off  block_type=inv
  Logical : xbsIndex_r4/Logical  logical_function=AND  inputs=2  en=off  latency=0  precision=Full  arith_type=Unsigned  n_bits=16  bin_pt=0  align_bp=on  dbl_ovrd=off  block_type=logical
      settings: block_version=11.4
  Terminator : Terminator
  gbe2 : xps_library/ten_GbE  Tag=xps:tengbe  SystemSampleTime=-1  port=ROACH:2  mac_lite=off  open_phy=off  show_param=off  pre_emph=3  swing=800  fab_en=off  fab_mac=0  fab_ip=0  fab_udp=0  fab_gate=0
  mcnt : Subsystem
  tag scope: gbe_gpu_led_rx=global, gbe_gpu_led_tx=global, gbe_gpu_led_up=global, gbe_gpu_rx_data=global, gbe_gpu_rx_eof=global, gbe_gpu_rx_ip=global, gbe_gpu_rx_port=global, gbe_gpu_rx_size=global, gbe_gpu_rx_valid=global, gbe_gpu_tx_ack=global
  wires:
  In2/1 -> Terminator/1
  [lb_mux_data] -> Delay/1
  [lb_mux_valid] -> Delay1/1
  [gpu_ip] -> gbe2/4
  [gpu_port] -> gbe2/5
  Delay/1 -> gbe2/2
  [gbe_gpu_rst] -> gbe2/1
  Constant1/1 -> gbe2/8, gbe2/7
  Delay10/1 -> gbe2/6
  gbe2/1 -> [gbe_gpu_led_up]
  gbe2/2 -> [gbe_gpu_led_rx]
  [gbe_gpu_disable] -> Inverter/1
  Inverter/1 -> Logical/1
  Logical/1 -> 10gbetxsnap/2(valid_in), gbe2/3
  gbe2/3 -> [gbe_gpu_led_tx]
  gbe2/4 -> [gbe_gpu_tx_ack]
  gbe2/5 -> [gbe_gpu_rx_data]
  gbe2/6 -> [gbe_gpu_rx_valid]
  gbe2/10 -> [gbe_gpu_rx_size]
  gbe2/7 -> [gbe_gpu_rx_ip]
  gbe2/8 -> [gbe_gpu_rx_port]
  gbe2/9 -> [gbe_gpu_rx_eof]
  Delay1/1 -> Logical/2
  [lb_mux_eof] -> Delay10/1
  Delay5/1 -> 10gbetxsnap/4(eof_in)
  [gbe_gpu_led_up] -> 10gbetxsnap/5(link_up)
  [gbe_gpu_led_tx] -> 10gbetxsnap/6(led_tx)
  [gbe_gpu_tx_ack] -> 10gbetxsnap/7(tx_ack)
  [gbe_gpu_tx_ack] -> Inverter1/1
  Inverter1/1 -> 10gbetxsnap/8(tx_notack)
  Delay3/1 -> 10gbetxsnap/1(data_in)
  [lb_mux_data] -> Delay3/1
  Constant2/1 -> Delay4/1
  Delay4/1 -> 10gbetxsnap/3(ip_in)
  [lb_mux_eof] -> Delay5/1
  [lb_mux_header] -> mcnt/2(header_valid)
  [lb_mux_data] -> mcnt/1(data)

== system roachf_2048_bak/input ==
  BitBasher : xbsIndex_r4/BitBasher  bitexpr=|  display_expr=off  sr_1=1  arith_type1=Unsigned  bin_pt1=0  sr_2=2  arith_type2=Unsigned  bin_pt2=0  sr_3=3  arith_type3=Unsigned  bin_pt3=0  sr_4=4  arith_type4=Unsigned  bin_pt4=0  dbl_ovrd=off  block_type=bitbasher
      | <html><head><meta name="qrichtext" content="1" /><style type="text/css">
      | p, li { white-space: pre-wrap; }
      | </style></head><body style=" font-family:'MS Shell Dlg 2'; font-size:8.25pt; font-weight:400; font-style:normal;">
      | <p style=" margin-top:12px; margin-bottom:12px; margin-left:0px; margin-right:0px; -qt-block-indent:0; text-indent:0px; font-family:'MS Shell Dlg'; font-size:8pt;">insel0={insel[1:0]}<br />insel1={insel[5:4]}<br />insel2={insel[9:8]}<br />insel3={insel[13:12]}</p></body></html>
      settings: block_version=10.1.3
  BitBasher1 : xbsIndex_r4/BitBasher  bitexpr=|  display_expr=off  sr_1=1  arith_type1=Unsigned  bin_pt1=0  sr_2=2  arith_type2=Unsigned  bin_pt2=0  sr_3=3  arith_type3=Unsigned  bin_pt3=0  sr_4=4  arith_type4=Unsigned  bin_pt4=0  dbl_ovrd=off  block_type=bitbasher
      | <html><head><meta name="qrichtext" content="1" /><style type="text/css">
      | p, li { white-space: pre-wrap; }
      | </style></head><body style=" font-family:'MS Shell Dlg 2'; font-size:8.25pt; font-weight:400; font-style:normal;">
      | <p style=" margin-top:12px; margin-bottom:12px; margin-left:0px; margin-right:0px; -qt-block-indent:0; text-indent:0px; font-family:'MS Shell Dlg'; font-size:8pt;">insel4={insel[17:16]}<br />insel5={insel[21:20]}<br />insel6={insel[25:24]}<br />insel7={insel[29:28]}</p></body></html>
      settings: block_version=10.1.3
  selector : xps_library/software register  Tag=xps:sw_reg  SystemSampleTime=-1  io_dir=From Processor  arith_type=Unsigned  bitwidth=32  bin_pt=0  sample_period=1  gw_name=roachf_2048_bak_input_selector_user_data_out
  tag scope: insel0=global, insel1=global, insel2=global, insel3=global, insel4=global, insel5=global, insel6=global, insel7=global
  wires:
  selector/1 -> BitBasher1/1, BitBasher/1
  BitBasher1/4 -> [insel7]
  BitBasher1/3 -> [insel6]
  BitBasher1/2 -> [insel5]
  BitBasher1/1 -> [insel4]
  BitBasher/4 -> [insel3]
  BitBasher/3 -> [insel2]
  BitBasher/2 -> [insel1]
  BitBasher/1 -> [insel0]

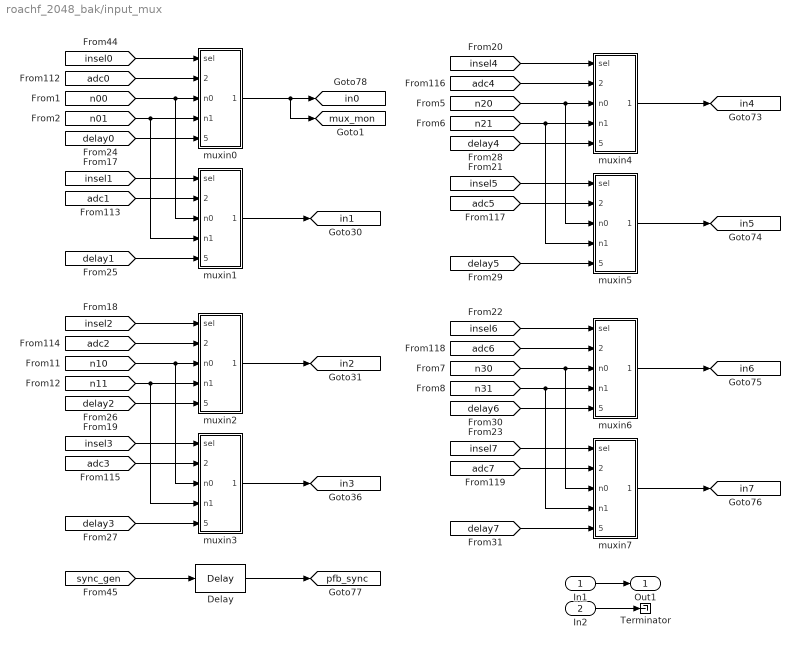
== system roachf_2048_bak/input_mux ==
  In1 : Inport
  In2 : Inport  Port=2
  Delay : xbsIndex_r4/Delay  rst=off  infoeditControl=Selection of Reset will increase slice count due to use of real FFs and instead of SRLs  en=off  latency=2  dbl_ovrd=off  reg_retiming=off  block_type=delay
  Terminator : Terminator
  muxin0 : Subsystem
  muxin1 : Subsystem
  muxin2 : Subsystem
  muxin3 : Subsystem
  muxin4 : Subsystem
  muxin5 : Subsystem
  muxin6 : Subsystem
  muxin7 : Subsystem
  Out1 : Outport
  tag scope: in0=global, in1=global, in2=global, in3=global, in4=global, in5=global, in6=global, in7=global, mux_mon=global, pfb_sync=global
  wires:
  [insel0] -> muxin0/1(sel)
  [adc0] -> muxin0/2(adc)
  [n00] -> muxin0/3(n0), muxin1/3(n0)
  [n01] -> muxin0/4(n1), muxin1/4(n1)
  [delay0] -> muxin0/5(delay)
  muxin0/1(out) -> [in0], [mux_mon]
  [insel1] -> muxin1/1(sel)
  [adc1] -> muxin1/2(adc)
  [delay1] -> muxin1/5(delay)
  [insel2] -> muxin2/1(sel)
  [adc2] -> muxin2/2(adc)
  [n10] -> muxin3/3(n0), muxin2/3(n0)
  [n11] -> muxin3/4(n1), muxin2/4(n1)
  [delay2] -> muxin2/5(delay)
  [insel3] -> muxin3/1(sel)
  [adc3] -> muxin3/2(adc)
  [delay3] -> muxin3/5(delay)
  muxin1/1(out) -> [in1]
  muxin2/1(out) -> [in2]
  muxin3/1(out) -> [in3]
  [insel4] -> muxin4/1(sel)
  [adc4] -> muxin4/2(adc)
  [n20] -> muxin5/3(n0), muxin4/3(n0)
  [n21] -> muxin5/4(n1), muxin4/4(n1)
  [delay4] -> muxin4/5(delay)
  [insel5] -> muxin5/1(sel)
  [adc5] -> muxin5/2(adc)
  [delay5] -> muxin5/5(delay)
  [insel6] -> muxin6/1(sel)
  [adc6] -> muxin6/2(adc)
  [n30] -> muxin7/3(n0), muxin6/3(n0)
  [n31] -> muxin7/4(n1), muxin6/4(n1)
  [delay6] -> muxin6/5(delay)
  [insel7] -> muxin7/1(sel)
  [adc7] -> muxin7/2(adc)
  [delay7] -> muxin7/5(delay)
  muxin7/1(out) -> [in7]
  muxin4/1(out) -> [in4]
  muxin5/1(out) -> [in5]
  muxin6/1(out) -> [in6]
  [sync_gen] -> Delay/1
  Delay/1 -> [pfb_sync]
  In1/1 -> Out1/1
  In2/1 -> Terminator/1
